simulink model "AcasXuClosedLoop_all_comp2017b"  (Simulink 9.0)
settings: Solver=VariableStepAuto  StartTime=0.0  StopTime=20  RelTol=1e-3
libraries: neural x14040, simulink x2
(part 38 of 534)

== system AcasXuClosedLoop_all_comp2017b/networks/Networks_m_9/AcasXu_3_9/Process Output 1 ==
  a : Inport  PortDimensions=5
      mask Display: e = 0.06;c1 = [120 230 180]/255;c2 = ([120 230 180]+0)/(256+128);c3 = ([120 230 180]+128)/(256+128);patch([0 1 1 0],[0 0 1 1],c1);patch([0 1 1 1-e 1-e 0+e],[0 0 1 1-e 0+e 0+e],c2);patch([0 0 1 1-e 0+e 0+e],[0 1 1 1-e 1-e 0+e],c3);plot([0 1 1 0 0],[0 0 1 1 0]);
  y : Outport  PortDimensions=5
      mask Display: e = 0.06;c1 = [120 230 180]/255;c2 = ([120 230 180]+0)/(256+128);c3 = ([120 230 180]+128)/(256+128);patch([0 1 1 0],[0 0 1 1],c1);patch([0 1 1 1-e 1-e 0+e],[0 0 1 1-e 0+e 0+e],c2);patch([0 0 1 1-e 0+e 0+e],[0 1 1 1-e 1-e 0+e],c3);plot([0 1 1 0 0],[0 0 1 1 0]);
  wires:
  a/1 -> y/1

== system AcasXuClosedLoop_all_comp2017b/networks/Networks_m_9/AcasXu_4_9/Layer 1 ==
  "p{1}" : Inport  PortDimensions=5
      mask Display: e = 0.06;c1 = [120 230 180]/255;c2 = ([120 230 180]+0)/(256+128);c3 = ([120 230 180]+128)/(256+128);patch([0 1 1 0],[0 0 1 1],c1);patch([0 1 1 1-e 1-e 0+e],[0 0 1 1-e 0+e 0+e],c2);patch([0 0 1 1-e 0+e 0+e],[0 1 1 1-e 1-e 0+e],c3);plot([0 1 1 0 0],[0 0 1 1 0]);
  Delays 1 : Subsystem
      mask Display: e = 0.06;c1 = [120 150 230]/255;c2 = ([120 150 230]+0)/383;c3 = ([120 150 230]+128)/383;patch([0 1 1 0],[0 0 1 1],c1);patch([0 1 1 1-e 1-e 0+e],[0 0 1 1-e 0+e 0+e],c2);patch([0 0 1 1-e 0+e 0+e],[0 1 1 1-e 1-e 0+e],c3);plot([0 1 1 0 0],[0 0 1 1 0]);disp('0');
  "IW{1,1}" : Subsystem
      mask Display: e = 0.06;c1 = [120 150 230]/255;c2 = ([120 150 230]+0)/383;c3 = ([120 150 230]+128)/383;patch([0 1 1 0],[0 0 1 1],c1);patch([0 1 1 1-e 1-e 0+e],[0 0 1 1-e 0+e 0+e],c2);patch([0 0 1 1-e 0+e 0+e],[0 1 1 1-e 1-e 0+e],c3);plot([0 1 1 0 0],[0 0 1 1 0]);disp('W');
  "b{1}" : Constant  Value=[-0.6222280000000000033111291486420668661594390869140625;-0.7286479999999999623838675688602961599826812744140625;-0.1096229999999999982218668037603492848575115203857421875;0.57803999999999999825917029738775454461574554443359375;0.1547759999999999969144681699617649428546428680419921875;-0.59774099999999996679633795793051831424236297607421875;-0.414026999999999978374631837141350843012332916259765625;-0.057743500000000003158362460453645326197147369384765625;-0.384350000000000024957813593573519028723239898681640625;-0.124423000000000005815792292196420021355152130126953125;-0.1039690000000000058566484995026257820427417755126953125;-0.40556999999999998607336237910203635692596435546875;0.13983000000000000984101689027738757431507110595703125;-0.321711000000000024723334490772685967385768890380859375;-0.27227699999999999125321892279316671192646026611328125;-0.0582171999999999967290165159283787943422794342041015625;0.0781797999999999937426053975286777131259441375732421875;0.1547659999999999869135791641383548267185688018798828125;-0.259782000000000012907008795082219876348972320556640625;-0.219667000000000001147526518252561800181865692138671875;-0.0356213999999999975099029825287288986146450042724609375;-0.287955000000000016502355038028326816856861114501953125;-0.041210900000000001697220142204969306476414203643798828125;-0.32555800000000001404032445861957967281341552734375;-0.509692999999999951654672258882783353328704833984375;-0.481935999999999975518250039385748095810413360595703125;0.05832880000000000009219291996487299911677837371826171875;-0.0853672000000000041897152414094307459890842437744140625;-0.1696100000000000107558406625685165636241436004638671875;0.2366870000000000084927620491725974716246128082275390625;-0.0724772999999999945064388384707854129374027252197265625;0.04211489999999999678248485679432633332908153533935546875;0.12322800000000000419930756834219209849834442138671875;0.1397259999999999890984980766006628982722759246826171875;-0.338374000000000008103739901343942619860172271728515625;-0.049813900000000001233591007121503935195505619049072265625;-0.490978999999999998760102926098625175654888153076171875;-0.03533540000000000291802138008279143832623958587646484375;-0.31175900000000000833466629046597518026828765869140625;0.0022653399999999998017108371328731664107181131839752197265625;-0.5714989999999999792379412610898725688457489013671875;0.072080599999999994675903280949569307267665863037109375;-0.05229720000000000201811900524262455292046070098876953125;0.2396560000000000079101170058493153192102909088134765625;0.9467330000000000467252903035841882228851318359375;-0.06532010000000000593534110748805687762796878814697265625;-0.475136999999999976029840809133020229637622833251953125;-0.52941099999999996494892684495425783097743988037109375;-0.39539000000000001922018100231071002781391143798828125;-0.1320530000000000037108094375071232207119464874267578125]
      mask Display: e = 0.06;c1 = [120 150 230]/255;c2 = ([120 150 230]+0)/383;c3 = ([120 150 230]+128)/383;patch([0 1 1 0],[0 0 1 1],c1);patch([0 1 1 1-e 1-e 0+e],[0 0 1 1-e 0+e 0+e],c2);patch([0 0 1 1-e 0+e 0+e],[0 1 1 1-e 1-e 0+e],c3);plot([0 1 1 0 0],[0 0 1 1 0]);disp('b');
  netsum : Sum  Inputs=2  SaturateOnIntegerOverflow=on  InputSameDT=on  OutDataTypeStr=Inherit: Same as first input
      mask Display: e = 0.06;c1 = [120 150 230]/255;c2 = ([120 150 230]+0)/383;c3 = ([120 150 230]+128)/383;patch([0 1 1 0],[0 0 1 1],c1);patch([0 1 1 1-e 1-e 0+e],[0 0 1 1-e 0+e 0+e],c2);patch([0 0 1 1-e 0+e 0+e],[0 1 1 1-e 1-e 0+e],c3);plot([0 1 1 0 0],[0 0 1 1 0]);disp('+');
  poslin : neural/Transfer Functions/poslin
      settings: SourceProductName=Deep Learning Toolbox  SourceProductBaseCode=NN
  "a{1}" : Outport  InitialOutput=[0;0;0;0;0;0;0;0;0;0;0;0;0;0;0;0;0;0;0;0;0;0;0;0;0;0;0;0;0;0;0;0;0;0;0;0;0;0;0;0;0;0;0;0;0;0;0;0;0;0]
      mask Display: e = 0.06;c1 = [120 230 180]/255;c2 = ([120 230 180]+0)/(256+128);c3 = ([120 230 180]+128)/(256+128);patch([0 1 1 0],[0 0 1 1],c1);patch([0 1 1 1-e 1-e 0+e],[0 0 1 1-e 0+e 0+e],c2);patch([0 0 1 1-e 0+e 0+e],[0 1 1 1-e 1-e 0+e],c3);plot([0 1 1 0 0],[0 0 1 1 0]);
  wires:
  poslin/1 -> "a{1}"/1
  netsum/1 -> poslin/1
  "b{1}"/1 -> netsum/2
  "IW{1,1}"/1("iz{1,1}") -> netsum/1
  Delays 1/1("pd{1,1}") -> "IW{1,1}"/1("pd{1,1}")
  "p{1}"/1 -> Delays 1/1("p{1}")

== system AcasXuClosedLoop_all_comp2017b/networks/Networks_m_9/AcasXu_4_9/Layer 2 ==
  "a{1} " : Inport  PortDimensions=50
      mask Display: e = 0.06;c1 = [120 230 180]/255;c2 = ([120 230 180]+0)/(256+128);c3 = ([120 230 180]+128)/(256+128);patch([0 1 1 0],[0 0 1 1],c1);patch([0 1 1 1-e 1-e 0+e],[0 0 1 1-e 0+e 0+e],c2);patch([0 0 1 1-e 0+e 0+e],[0 1 1 1-e 1-e 0+e],c3);plot([0 1 1 0 0],[0 0 1 1 0]);
  Delays 1 : Subsystem
      mask Display: e = 0.06;c1 = [120 150 230]/255;c2 = ([120 150 230]+0)/383;c3 = ([120 150 230]+128)/383;patch([0 1 1 0],[0 0 1 1],c1);patch([0 1 1 1-e 1-e 0+e],[0 0 1 1-e 0+e 0+e],c2);patch([0 0 1 1-e 0+e 0+e],[0 1 1 1-e 1-e 0+e],c3);plot([0 1 1 0 0],[0 0 1 1 0]);disp('0');
  "LW{2,1}" : Subsystem
      mask Display: e = 0.06;c1 = [120 150 230]/255;c2 = ([120 150 230]+0)/383;c3 = ([120 150 230]+128)/383;patch([0 1 1 0],[0 0 1 1],c1);patch([0 1 1 1-e 1-e 0+e],[0 0 1 1-e 0+e 0+e],c2);patch([0 0 1 1-e 0+e 0+e],[0 1 1 1-e 1-e 0+e],c3);plot([0 1 1 0 0],[0 0 1 1 0]);disp('W');
  "b{2}" : Constant  Value=[1.02522000000000002017941369558684527873992919921875;-0.562000999999999972800424075103364884853363037109375;-0.00946180999999999931826533128287337603978812694549560546875;0.17765500000000000735411731511703692376613616943359375;0.79179600000000005532996283363900147378444671630859375;-0.5709340000000000525659515915322117507457733154296875;-0.0059997399999999999342659151579937315545976161956787109375;-0.32771099999999997454125377771561034023761749267578125;-0.7948239999999999749746848465292714536190032958984375;0.76970400000000005480416120917652733623981475830078125;-0.0251702000000000002122302333873449242673814296722412109375;1.61854999999999993320898283855058252811431884765625;-0.3540360000000000173514536072616465389728546142578125;-0.00907804000000000076153749972718287608586251735687255859375;-0.0709912000000000042998493654522462747991085052490234375;0.368391999999999997239541471572010777890682220458984375;0.272660999999999986709298127607326023280620574951171875;-0.284345000000000014406253967536031268537044525146484375;-0.352752000000000009993783578465809114277362823486328125;0.96926900000000004720135393654345534741878509521484375;2.320460000000000189146476259338669478893280029296875;0.4902889999999999748325762993772514164447784423828125;-0.342196000000000000174082970261224545538425445556640625;0.398270999999999986140863938999245874583721160888671875;-0.016967400000000000537259126076605753041803836822509765625;0.331542999999999976612485852456302382051944732666015625;-0.06554050000000000153477230924181640148162841796875;0.4595569999999999932782657197094522416591644287109375;-1.162080000000000001847411112976260483264923095703125;-0.400434000000000012153833495176513679325580596923828125;0.55777299999999996327204598856042139232158660888671875;-0.291293000000000024130031306413002312183380126953125;-0.0113866000000000001934896687316722818650305271148681640625;-0.53071699999999999430855268656159751117229461669921875;-0.2219279999999999863913302533546811901032924652099609375;-0.015296099999999999974331643670666380785405635833740234375;-0.0083213399999999999867572597622711327858269214630126953125;-0.2919359999999999732978039901354350149631500244140625;-0.50425200000000003353051170051912777125835418701171875;-0.31965399999999999369748593380791135132312774658203125;1.0295199999999999906918901615426875650882720947265625;-0.75094000000000005190514684727531857788562774658203125;-0.45472400000000001707434194031520746648311614990234375;-0.01299699999999999987243537447056951350532472133636474609375;0.1546980000000000021742607714259065687656402587890625;-0.67860200000000003850431085083982907235622406005859375;-0.57151099999999999123900806807796470820903778076171875;-0.019285099999999999464961319972644560039043426513671875;-0.4202799999999999869260136620141565799713134765625;-0.52828500000000000458300064565264619886875152587890625]
      mask Display: e = 0.06;c1 = [120 150 230]/255;c2 = ([120 150 230]+0)/383;c3 = ([120 150 230]+128)/383;patch([0 1 1 0],[0 0 1 1],c1);patch([0 1 1 1-e 1-e 0+e],[0 0 1 1-e 0+e 0+e],c2);patch([0 0 1 1-e 0+e 0+e],[0 1 1 1-e 1-e 0+e],c3);plot([0 1 1 0 0],[0 0 1 1 0]);disp('b');
  netsum : Sum  Inputs=2  SaturateOnIntegerOverflow=on  InputSameDT=on  OutDataTypeStr=Inherit: Same as first input
      mask Display: e = 0.06;c1 = [120 150 230]/255;c2 = ([120 150 230]+0)/383;c3 = ([120 150 230]+128)/383;patch([0 1 1 0],[0 0 1 1],c1);patch([0 1 1 1-e 1-e 0+e],[0 0 1 1-e 0+e 0+e],c2);patch([0 0 1 1-e 0+e 0+e],[0 1 1 1-e 1-e 0+e],c3);plot([0 1 1 0 0],[0 0 1 1 0]);disp('+');
  poslin : neural/Transfer Functions/poslin
      settings: SourceProductName=Deep Learning Toolbox  SourceProductBaseCode=NN
  "a{2}" : Outport  InitialOutput=[0;0;0;0;0;0;0;0;0;0;0;0;0;0;0;0;0;0;0;0;0;0;0;0;0;0;0;0;0;0;0;0;0;0;0;0;0;0;0;0;0;0;0;0;0;0;0;0;0;0]
      mask Display: e = 0.06;c1 = [120 230 180]/255;c2 = ([120 230 180]+0)/(256+128);c3 = ([120 230 180]+128)/(256+128);patch([0 1 1 0],[0 0 1 1],c1);patch([0 1 1 1-e 1-e 0+e],[0 0 1 1-e 0+e 0+e],c2);patch([0 0 1 1-e 0+e 0+e],[0 1 1 1-e 1-e 0+e],c3);plot([0 1 1 0 0],[0 0 1 1 0]);
  wires:
  poslin/1 -> "a{2}"/1
  netsum/1 -> poslin/1
  "b{2}"/1 -> netsum/2
  "LW{2,1}"/1("lz{2,1}") -> netsum/1
  Delays 1/1("pd{2,1}") -> "LW{2,1}"/1("ad{2,1}")
  "a{1} "/1 -> Delays 1/1("p{2}")

== system AcasXuClosedLoop_all_comp2017b/networks/Networks_m_9/AcasXu_4_9/Layer 3 ==
  "a{2} " : Inport  PortDimensions=50
      mask Display: e = 0.06;c1 = [120 230 180]/255;c2 = ([120 230 180]+0)/(256+128);c3 = ([120 230 180]+128)/(256+128);patch([0 1 1 0],[0 0 1 1],c1);patch([0 1 1 1-e 1-e 0+e],[0 0 1 1-e 0+e 0+e],c2);patch([0 0 1 1-e 0+e 0+e],[0 1 1 1-e 1-e 0+e],c3);plot([0 1 1 0 0],[0 0 1 1 0]);
  Delays 1 : Subsystem
      mask Display: e = 0.06;c1 = [120 150 230]/255;c2 = ([120 150 230]+0)/383;c3 = ([120 150 230]+128)/383;patch([0 1 1 0],[0 0 1 1],c1);patch([0 1 1 1-e 1-e 0+e],[0 0 1 1-e 0+e 0+e],c2);patch([0 0 1 1-e 0+e 0+e],[0 1 1 1-e 1-e 0+e],c3);plot([0 1 1 0 0],[0 0 1 1 0]);disp('0');
  "LW{3,2}" : Subsystem
      mask Display: e = 0.06;c1 = [120 150 230]/255;c2 = ([120 150 230]+0)/383;c3 = ([120 150 230]+128)/383;patch([0 1 1 0],[0 0 1 1],c1);patch([0 1 1 1-e 1-e 0+e],[0 0 1 1-e 0+e 0+e],c2);patch([0 0 1 1-e 0+e 0+e],[0 1 1 1-e 1-e 0+e],c3);plot([0 1 1 0 0],[0 0 1 1 0]);disp('W');
  "b{3}" : Constant  Value=[-0.1822640000000000093383079047271166928112506866455078125;0.62188699999999996759214582198183052241802215576171875;1.226879999999999970583530739531852304935455322265625;0.387351999999999974111375422580749727785587310791015625;-2.192489999999999827906549398903734982013702392578125;-0.341052999999999995051069845430902205407619476318359375;-2.135260000000000157882595885894261300563812255859375;-0.65622400000000002950883981611696071922779083251953125;-1.2105399999999999494093572138808667659759521484375;0.293040000000000022684076839141198433935642242431640625;-1.134489999999999998436805981327779591083526611328125;-2.153630000000000155324642037157900631427764892578125;-0.365439999999999987068122209166176617145538330078125;-0.97034399999999998431832182177458889782428741455078125;0.280422000000000004593658786689047701656818389892578125;-0.5748100000000000431299440606380812823772430419921875;-0.632265999999999994685140336514450609683990478515625;0.8990700000000000358113538823090493679046630859375;-2.217560000000000197672989088459871709346771240234375;1.2218299999999999716493448431720025837421417236328125;-1.4797000000000000152766688188421539962291717529296875;-0.47529399999999999426592012241599150002002716064453125;-0.89124099999999994992094798362813889980316162109375;-3.7400000000000002131628207280300557613372802734375;-1.6563499999999999889865875957184471189975738525390625;0.34078900000000000858335624798201024532318115234375;2.00767000000000006565414878423325717449188232421875;-0.073932100000000000594724269831203855574131011962890625;-2.95357000000000002870592652470804750919342041015625;-0.484140999999999988023802188763511367142200469970703125;0.21756100000000000438404867963981814682483673095703125;-0.276013999999999981582732289098203182220458984375;-2.63429000000000002046363078989088535308837890625;-0.783528000000000002245315045001916587352752685546875;-3.292660000000000142250655699172057211399078369140625;1.0612299999999998956212721168412826955318450927734375;0.51146100000000005447731155072688125073909759521484375;0.79009099999999998775734866285347379744052886962890625;0.90034000000000002916777930295211263000965118408203125;-0.99270000000000002682298827494378201663494110107421875;-1.1930099999999999038635678516584448516368865966796875;0.65531600000000000960653778747655451297760009765625;0.27336199999999999388222704510553739964962005615234375;-1.218409999999999993036681189551018178462982177734375;1.033630000000000048743231673142872750759124755859375;0.165285999999999988485654967007576487958431243896484375;0.333407999999999982154719191385083831846714019775390625;0.07858579999999999732285260733988252468407154083251953125;-0.11880100000000000382005538313023862428963184356689453125;0.458838000000000023614887822986929677426815032958984375]
      mask Display: e = 0.06;c1 = [120 150 230]/255;c2 = ([120 150 230]+0)/383;c3 = ([120 150 230]+128)/383;patch([0 1 1 0],[0 0 1 1],c1);patch([0 1 1 1-e 1-e 0+e],[0 0 1 1-e 0+e 0+e],c2);patch([0 0 1 1-e 0+e 0+e],[0 1 1 1-e 1-e 0+e],c3);plot([0 1 1 0 0],[0 0 1 1 0]);disp('b');
  netsum : Sum  Inputs=2  SaturateOnIntegerOverflow=on  InputSameDT=on  OutDataTypeStr=Inherit: Same as first input
      mask Display: e = 0.06;c1 = [120 150 230]/255;c2 = ([120 150 230]+0)/383;c3 = ([120 150 230]+128)/383;patch([0 1 1 0],[0 0 1 1],c1);patch([0 1 1 1-e 1-e 0+e],[0 0 1 1-e 0+e 0+e],c2);patch([0 0 1 1-e 0+e 0+e],[0 1 1 1-e 1-e 0+e],c3);plot([0 1 1 0 0],[0 0 1 1 0]);disp('+');
  poslin : neural/Transfer Functions/poslin
      settings: SourceProductName=Deep Learning Toolbox  SourceProductBaseCode=NN
  "a{3}" : Outport  InitialOutput=[0;0;0;0;0;0;0;0;0;0;0;0;0;0;0;0;0;0;0;0;0;0;0;0;0;0;0;0;0;0;0;0;0;0;0;0;0;0;0;0;0;0;0;0;0;0;0;0;0;0]
      mask Display: e = 0.06;c1 = [120 230 180]/255;c2 = ([120 230 180]+0)/(256+128);c3 = ([120 230 180]+128)/(256+128);patch([0 1 1 0],[0 0 1 1],c1);patch([0 1 1 1-e 1-e 0+e],[0 0 1 1-e 0+e 0+e],c2);patch([0 0 1 1-e 0+e 0+e],[0 1 1 1-e 1-e 0+e],c3);plot([0 1 1 0 0],[0 0 1 1 0]);
  wires:
  poslin/1 -> "a{3}"/1
  netsum/1 -> poslin/1
  "b{3}"/1 -> netsum/2
  "LW{3,2}"/1("lz{3,2}") -> netsum/1
  Delays 1/1("pd{3,2}") -> "LW{3,2}"/1("ad{3,2}")
  "a{2} "/1 -> Delays 1/1("p{3}")

== system AcasXuClosedLoop_all_comp2017b/networks/Networks_m_9/AcasXu_4_9/Layer 4 ==
  "a{3} " : Inport  PortDimensions=50
      mask Display: e = 0.06;c1 = [120 230 180]/255;c2 = ([120 230 180]+0)/(256+128);c3 = ([120 230 180]+128)/(256+128);patch([0 1 1 0],[0 0 1 1],c1);patch([0 1 1 1-e 1-e 0+e],[0 0 1 1-e 0+e 0+e],c2);patch([0 0 1 1-e 0+e 0+e],[0 1 1 1-e 1-e 0+e],c3);plot([0 1 1 0 0],[0 0 1 1 0]);
  Delays 1 : Subsystem
      mask Display: e = 0.06;c1 = [120 150 230]/255;c2 = ([120 150 230]+0)/383;c3 = ([120 150 230]+128)/383;patch([0 1 1 0],[0 0 1 1],c1);patch([0 1 1 1-e 1-e 0+e],[0 0 1 1-e 0+e 0+e],c2);patch([0 0 1 1-e 0+e 0+e],[0 1 1 1-e 1-e 0+e],c3);plot([0 1 1 0 0],[0 0 1 1 0]);disp('0');
  "LW{4,3}" : Subsystem
      mask Display: e = 0.06;c1 = [120 150 230]/255;c2 = ([120 150 230]+0)/383;c3 = ([120 150 230]+128)/383;patch([0 1 1 0],[0 0 1 1],c1);patch([0 1 1 1-e 1-e 0+e],[0 0 1 1-e 0+e 0+e],c2);patch([0 0 1 1-e 0+e 0+e],[0 1 1 1-e 1-e 0+e],c3);plot([0 1 1 0 0],[0 0 1 1 0]);disp('W');
  "b{4}" : Constant  Value=[-0.89365000000000005542233338928781449794769287109375;0.1854739999999999999769073610877967439591884613037109375;-1.8181799999999999073452272568829357624053955078125;2.8344100000000000960653778747655451297760009765625;-1.0596099999999999408117901111836545169353485107421875;-5.882780000000000342197381542064249515533447265625;-2.30323000000000011056044968427158892154693603515625;-0.78521300000000004981615120414062403142452239990234375;-2.105259999999999909192638369859196245670318603515625;-2.711160000000000014352963262354023754596710205078125;1.9786099999999999798916405779891647398471832275390625;-1.0117499999999999271693695845897309482097625732421875;2.79769999999999985362819643341936171054840087890625;0.2040669999999999983941734171821735799312591552734375;-0.380328999999999972647657386914943344891071319580078125;-0.006045140000000000062019278601610494661144912242889404296875;0.06022650000000000225508500761861796490848064422607421875;-0.05921819999999999861728383621084503829479217529296875;5.17250999999999994116706147906370460987091064453125;1.5604800000000000892441676114685833454132080078125;1.4627499999999999946709294817992486059665679931640625;-2.630640000000000089386276158620603382587432861328125;1.65083000000000001961097950697876513004302978515625;-2.36413999999999990819787853979505598545074462890625;0.146766000000000007563727422166266478598117828369140625;-1.598829999999999973425701682572253048419952392578125;-0.065557699999999996531840906754950992763042449951171875;-2.61073999999999983856469043530523777008056640625;2.0779399999999998982502802391536533832550048828125;-1.2690300000000001023892082230304367840290069580078125;-0.1732309999999999960973440238376497291028499603271484375;3.5928100000000000591171556152403354644775390625;-2.93836999999999992638777257525362074375152587890625;-1.4845600000000001017497197608463466167449951171875;1.0714900000000000535038680027355439960956573486328125;-5.20971999999999990649257597397081553936004638671875;1.764180000000000081428197518107481300830841064453125;-2.023140000000000160440549734630621969699859619140625;-0.54592300000000004711608880825224332511425018310546875;-1.1615599999999999258903926602215506136417388916015625;-0.343324000000000018051338201985345222055912017822265625;-0.0765071999999999974306774674914777278900146484375;-2.868729999999999780158077555825002491474151611328125;-2.16922000000000014807710613240487873554229736328125;0.034253999999999999725996957522511365823447704315185546875;-5.417989999999999639612724422477185726165771484375;-2.7816700000000000869704308570362627506256103515625;-2.69573000000000018161472326028160750865936279296875;-1.645869999999999944151340969256125390529632568359375;-0.041442600000000003046540797413399559445679187774658203125]
      mask Display: e = 0.06;c1 = [120 150 230]/255;c2 = ([120 150 230]+0)/383;c3 = ([120 150 230]+128)/383;patch([0 1 1 0],[0 0 1 1],c1);patch([0 1 1 1-e 1-e 0+e],[0 0 1 1-e 0+e 0+e],c2);patch([0 0 1 1-e 0+e 0+e],[0 1 1 1-e 1-e 0+e],c3);plot([0 1 1 0 0],[0 0 1 1 0]);disp('b');
  netsum : Sum  Inputs=2  SaturateOnIntegerOverflow=on  InputSameDT=on  OutDataTypeStr=Inherit: Same as first input
      mask Display: e = 0.06;c1 = [120 150 230]/255;c2 = ([120 150 230]+0)/383;c3 = ([120 150 230]+128)/383;patch([0 1 1 0],[0 0 1 1],c1);patch([0 1 1 1-e 1-e 0+e],[0 0 1 1-e 0+e 0+e],c2);patch([0 0 1 1-e 0+e 0+e],[0 1 1 1-e 1-e 0+e],c3);plot([0 1 1 0 0],[0 0 1 1 0]);disp('+');
  poslin : neural/Transfer Functions/poslin
      settings: SourceProductName=Deep Learning Toolbox  SourceProductBaseCode=NN
  "a{4}" : Outport  InitialOutput=[0;0;0;0;0;0;0;0;0;0;0;0;0;0;0;0;0;0;0;0;0;0;0;0;0;0;0;0;0;0;0;0;0;0;0;0;0;0;0;0;0;0;0;0;0;0;0;0;0;0]
      mask Display: e = 0.06;c1 = [120 230 180]/255;c2 = ([120 230 180]+0)/(256+128);c3 = ([120 230 180]+128)/(256+128);patch([0 1 1 0],[0 0 1 1],c1);patch([0 1 1 1-e 1-e 0+e],[0 0 1 1-e 0+e 0+e],c2);patch([0 0 1 1-e 0+e 0+e],[0 1 1 1-e 1-e 0+e],c3);plot([0 1 1 0 0],[0 0 1 1 0]);
  wires:
  poslin/1 -> "a{4}"/1
  netsum/1 -> poslin/1
  "b{4}"/1 -> netsum/2
  "LW{4,3}"/1("lz{4,3}") -> netsum/1
  Delays 1/1("pd{4,3}") -> "LW{4,3}"/1("ad{4,3}")
  "a{3} "/1 -> Delays 1/1("p{4}")

== system AcasXuClosedLoop_all_comp2017b/networks/Networks_m_9/AcasXu_4_9/Layer 5 ==
  "a{4} " : Inport  PortDimensions=50
      mask Display: e = 0.06;c1 = [120 230 180]/255;c2 = ([120 230 180]+0)/(256+128);c3 = ([120 230 180]+128)/(256+128);patch([0 1 1 0],[0 0 1 1],c1);patch([0 1 1 1-e 1-e 0+e],[0 0 1 1-e 0+e 0+e],c2);patch([0 0 1 1-e 0+e 0+e],[0 1 1 1-e 1-e 0+e],c3);plot([0 1 1 0 0],[0 0 1 1 0]);
  Delays 1 : Subsystem
      mask Display: e = 0.06;c1 = [120 150 230]/255;c2 = ([120 150 230]+0)/383;c3 = ([120 150 230]+128)/383;patch([0 1 1 0],[0 0 1 1],c1);patch([0 1 1 1-e 1-e 0+e],[0 0 1 1-e 0+e 0+e],c2);patch([0 0 1 1-e 0+e 0+e],[0 1 1 1-e 1-e 0+e],c3);plot([0 1 1 0 0],[0 0 1 1 0]);disp('0');
  "LW{5,4}" : Subsystem
      mask Display: e = 0.06;c1 = [120 150 230]/255;c2 = ([120 150 230]+0)/383;c3 = ([120 150 230]+128)/383;patch([0 1 1 0],[0 0 1 1],c1);patch([0 1 1 1-e 1-e 0+e],[0 0 1 1-e 0+e 0+e],c2);patch([0 0 1 1-e 0+e 0+e],[0 1 1 1-e 1-e 0+e],c3);plot([0 1 1 0 0],[0 0 1 1 0]);disp('W');
  "b{5}" : Constant  Value=[-0.0191056999999999997663646666978820576332509517669677734375;1.3559200000000000141398004416259936988353729248046875;-0.71357999999999999207744849627488292753696441650390625;-8.3485700000000004905587047687731683254241943359375;-1.559309999999999973852027324028313159942626953125;2.3629400000000000403588273911736905574798583984375;0.51666100000000003689137884066440165042877197265625;0.468301000000000022804869104220415465533733367919921875;-0.261516000000000026215474235868896357715129852294921875;3.70849999999999990762944435118697583675384521484375;-0.89031599999999999628386149197467602789402008056640625;-0.026237400000000000999111904320670873858034610748291015625;0.19159599999999998853894567218958400189876556396484375;1.3666899999999999604227696181624196469783782958984375;2.403910000000000213304929275182075798511505126953125;2.186739999999999906066250332514755427837371826171875;-0.76852200000000003843325657726381905376911163330078125;0.315346999999999988428811548146768473088741302490234375;-0.87672000000000005481837206389172933995723724365234375;-1.57667000000000001591615728102624416351318359375;0.6877590000000000092228447101661004126071929931640625;-5.07626000000000043854697651113383471965789794921875;0.9768879999999999785842419441905803978443145751953125;-0.9757780000000000342197381542064249515533447265625;1.7846100000000000296296320811961777508258819580078125;4.19756999999999980133225108147598803043365478515625;-1.7266799999999999926103555480949580669403076171875;-1.93334999999999990194510246510617434978485107421875;-1.4522500000000000408562073062057606875896453857421875;-0.29601800000000000334665628543007187545299530029296875;-3.774789999999999867696942601469345390796661376953125;-0.189578999999999997516653138518449850380420684814453125;1.0545700000000000073896444519050419330596923828125;-0.11671499999999999930277994053540169261395931243896484375;2.40486999999999984112264428404159843921661376953125;4.52629000000000036862957131233997642993927001953125;2.59363000000000010203393685515038669109344482421875;3.37394000000000016115109247039072215557098388671875;1.9498800000000000576250158701441250741481781005859375;-2.4771499999999999630517777404747903347015380859375;-5.73190000000000043911541069974191486835479736328125;1.1379099999999999770494696349487639963626861572265625;-2.4970400000000001483613232267089188098907470703125;2.980239999999999778168557895696721971035003662109375;-0.66089600000000003898747991115669719874858856201171875;3.55039000000000015688783605583012104034423828125;-3.1092800000000000437694325228221714496612548828125;-0.27077600000000001667643800828955136239528656005859375;2.273099999999999898392388786305673420429229736328125;-1.494310000000000027142732506035827100276947021484375]
      mask Display: e = 0.06;c1 = [120 150 230]/255;c2 = ([120 150 230]+0)/383;c3 = ([120 150 230]+128)/383;patch([0 1 1 0],[0 0 1 1],c1);patch([0 1 1 1-e 1-e 0+e],[0 0 1 1-e 0+e 0+e],c2);patch([0 0 1 1-e 0+e 0+e],[0 1 1 1-e 1-e 0+e],c3);plot([0 1 1 0 0],[0 0 1 1 0]);disp('b');
  netsum : Sum  Inputs=2  SaturateOnIntegerOverflow=on  InputSameDT=on  OutDataTypeStr=Inherit: Same as first input
      mask Display: e = 0.06;c1 = [120 150 230]/255;c2 = ([120 150 230]+0)/383;c3 = ([120 150 230]+128)/383;patch([0 1 1 0],[0 0 1 1],c1);patch([0 1 1 1-e 1-e 0+e],[0 0 1 1-e 0+e 0+e],c2);patch([0 0 1 1-e 0+e 0+e],[0 1 1 1-e 1-e 0+e],c3);plot([0 1 1 0 0],[0 0 1 1 0]);disp('+');
  poslin : neural/Transfer Functions/poslin
      settings: SourceProductName=Deep Learning Toolbox  SourceProductBaseCode=NN
  "a{5}" : Outport  InitialOutput=[0;0;0;0;0;0;0;0;0;0;0;0;0;0;0;0;0;0;0;0;0;0;0;0;0;0;0;0;0;0;0;0;0;0;0;0;0;0;0;0;0;0;0;0;0;0;0;0;0;0]
      mask Display: e = 0.06;c1 = [120 230 180]/255;c2 = ([120 230 180]+0)/(256+128);c3 = ([120 230 180]+128)/(256+128);patch([0 1 1 0],[0 0 1 1],c1);patch([0 1 1 1-e 1-e 0+e],[0 0 1 1-e 0+e 0+e],c2);patch([0 0 1 1-e 0+e 0+e],[0 1 1 1-e 1-e 0+e],c3);plot([0 1 1 0 0],[0 0 1 1 0]);
  wires:
  poslin/1 -> "a{5}"/1
  netsum/1 -> poslin/1
  "b{5}"/1 -> netsum/2
  "LW{5,4}"/1("lz{5,4}") -> netsum/1
  Delays 1/1("pd{5,4}") -> "LW{5,4}"/1("ad{5,4}")
  "a{4} "/1 -> Delays 1/1("p{5}")

== system AcasXuClosedLoop_all_comp2017b/networks/Networks_m_9/AcasXu_4_9/Layer 6 ==
  "a{5} " : Inport  PortDimensions=50
      mask Display: e = 0.06;c1 = [120 230 180]/255;c2 = ([120 230 180]+0)/(256+128);c3 = ([120 230 180]+128)/(256+128);patch([0 1 1 0],[0 0 1 1],c1);patch([0 1 1 1-e 1-e 0+e],[0 0 1 1-e 0+e 0+e],c2);patch([0 0 1 1-e 0+e 0+e],[0 1 1 1-e 1-e 0+e],c3);plot([0 1 1 0 0],[0 0 1 1 0]);
  Delays 1 : Subsystem
      mask Display: e = 0.06;c1 = [120 150 230]/255;c2 = ([120 150 230]+0)/383;c3 = ([120 150 230]+128)/383;patch([0 1 1 0],[0 0 1 1],c1);patch([0 1 1 1-e 1-e 0+e],[0 0 1 1-e 0+e 0+e],c2);patch([0 0 1 1-e 0+e 0+e],[0 1 1 1-e 1-e 0+e],c3);plot([0 1 1 0 0],[0 0 1 1 0]);disp('0');
  "LW{6,5}" : Subsystem
      mask Display: e = 0.06;c1 = [120 150 230]/255;c2 = ([120 150 230]+0)/383;c3 = ([120 150 230]+128)/383;patch([0 1 1 0],[0 0 1 1],c1);patch([0 1 1 1-e 1-e 0+e],[0 0 1 1-e 0+e 0+e],c2);patch([0 0 1 1-e 0+e 0+e],[0 1 1 1-e 1-e 0+e],c3);plot([0 1 1 0 0],[0 0 1 1 0]);disp('W');
  "b{6}" : Constant  Value=[3.47473000000000009634959496906958520412445068359375;-0.76460099999999997510968796632369048893451690673828125;-2.842560000000000197672989088459871709346771240234375;-5.66467000000000009407585821463726460933685302734375;0.121755000000000002113864638886298052966594696044921875;-0.2228239999999999942925654750069952569901943206787109375;1.6902399999999999646860260327230207622051239013671875;-2.8998200000000000642330633127130568027496337890625;2.301550000000000206767936106189154088497161865234375;0.8894349999999999756283841634285636246204376220703125;2.950919999999999987494447850622236728668212890625;3.1411899999999999266719896695576608180999755859375;3.9182100000000001926991899381391704082489013671875;5.5350500000000000255795384873636066913604736328125;-4.911590000000000344471118296496570110321044921875;3.93102999999999980218490236438810825347900390625;-2.903490000000000126334498418145813047885894775390625;-1.28774999999999995026200849679298698902130126953125;-2.450200000000000155608859131461940705776214599609375;-2.1671800000000001062971932697109878063201904296875;-6.09743000000000012761347534251399338245391845703125;-7.596199999999999619149093632586300373077392578125;-2.58457999999999987750243235495872795581817626953125;-0.2267530000000000101056940593480248935520648956298828125;-1.70751000000000008327560863108374178409576416015625;0.662668000000000034788172342814505100250244140625;3.66957000000000022055246517993509769439697265625;-2.662520000000000219841922444174997508525848388671875;1.6607600000000000139976918944739736616611480712890625;-2.701020000000000198525640371371991932392120361328125;4.69108000000000036067149267182685434818267822265625;-2.577240000000000197388771994155831634998321533203125;2.096670000000000033679725675028748810291290283203125;6.0103600000000003689137884066440165042877197265625;2.811970000000000080575546235195361077785491943359375;0.351059999999999983177900730879628099501132965087890625;1.62349999999999994315658113919198513031005859375;-2.54253000000000017877255231724120676517486572265625;0.0238302999999999987057464068129775114357471466064453125;0.12396400000000000474198458277896861545741558074951171875;2.445469999999999810569306646357290446758270263671875;-4.7998700000000003029754225281067192554473876953125;-2.496849999999999791810978422290645539760589599609375;1.4914099999999999024424823801382444798946380615234375;-4.76001000000000029643842935911379754543304443359375;0.66468899999999997429966924755717627704143524169921875;-3.36561999999999983401721692644059658050537109375;0.702080000000000037374547900981269776821136474609375;-2.528090000000000170388148035272024571895599365234375;-3.319780000000000175219838638440705835819244384765625]
      mask Display: e = 0.06;c1 = [120 150 230]/255;c2 = ([120 150 230]+0)/383;c3 = ([120 150 230]+128)/383;patch([0 1 1 0],[0 0 1 1],c1);patch([0 1 1 1-e 1-e 0+e],[0 0 1 1-e 0+e 0+e],c2);patch([0 0 1 1-e 0+e 0+e],[0 1 1 1-e 1-e 0+e],c3);plot([0 1 1 0 0],[0 0 1 1 0]);disp('b');
  netsum : Sum  Inputs=2  SaturateOnIntegerOverflow=on  InputSameDT=on  OutDataTypeStr=Inherit: Same as first input
      mask Display: e = 0.06;c1 = [120 150 230]/255;c2 = ([120 150 230]+0)/383;c3 = ([120 150 230]+128)/383;patch([0 1 1 0],[0 0 1 1],c1);patch([0 1 1 1-e 1-e 0+e],[0 0 1 1-e 0+e 0+e],c2);patch([0 0 1 1-e 0+e 0+e],[0 1 1 1-e 1-e 0+e],c3);plot([0 1 1 0 0],[0 0 1 1 0]);disp('+');
  poslin : neural/Transfer Functions/poslin
      settings: SourceProductName=Deep Learning Toolbox  SourceProductBaseCode=NN
  "a{6}" : Outport  InitialOutput=[0;0;0;0;0;0;0;0;0;0;0;0;0;0;0;0;0;0;0;0;0;0;0;0;0;0;0;0;0;0;0;0;0;0;0;0;0;0;0;0;0;0;0;0;0;0;0;0;0;0]
      mask Display: e = 0.06;c1 = [120 230 180]/255;c2 = ([120 230 180]+0)/(256+128);c3 = ([120 230 180]+128)/(256+128);patch([0 1 1 0],[0 0 1 1],c1);patch([0 1 1 1-e 1-e 0+e],[0 0 1 1-e 0+e 0+e],c2);patch([0 0 1 1-e 0+e 0+e],[0 1 1 1-e 1-e 0+e],c3);plot([0 1 1 0 0],[0 0 1 1 0]);
  wires:
  poslin/1 -> "a{6}"/1
  netsum/1 -> poslin/1
  "b{6}"/1 -> netsum/2
  "LW{6,5}"/1("lz{6,5}") -> netsum/1
  Delays 1/1("pd{6,5}") -> "LW{6,5}"/1("ad{6,5}")
  "a{5} "/1 -> Delays 1/1("p{6}")

== system AcasXuClosedLoop_all_comp2017b/networks/Networks_m_9/AcasXu_4_9/Layer 7 ==
  "a{6} " : Inport  PortDimensions=50
      mask Display: e = 0.06;c1 = [120 230 180]/255;c2 = ([120 230 180]+0)/(256+128);c3 = ([120 230 180]+128)/(256+128);patch([0 1 1 0],[0 0 1 1],c1);patch([0 1 1 1-e 1-e 0+e],[0 0 1 1-e 0+e 0+e],c2);patch([0 0 1 1-e 0+e 0+e],[0 1 1 1-e 1-e 0+e],c3);plot([0 1 1 0 0],[0 0 1 1 0]);
  Delays 1 : Subsystem
      mask Display: e = 0.06;c1 = [120 150 230]/255;c2 = ([120 150 230]+0)/383;c3 = ([120 150 230]+128)/383;patch([0 1 1 0],[0 0 1 1],c1);patch([0 1 1 1-e 1-e 0+e],[0 0 1 1-e 0+e 0+e],c2);patch([0 0 1 1-e 0+e 0+e],[0 1 1 1-e 1-e 0+e],c3);plot([0 1 1 0 0],[0 0 1 1 0]);disp('0');
  "LW{7,6}" : Subsystem
      mask Display: e = 0.06;c1 = [120 150 230]/255;c2 = ([120 150 230]+0)/383;c3 = ([120 150 230]+128)/383;patch([0 1 1 0],[0 0 1 1],c1);patch([0 1 1 1-e 1-e 0+e],[0 0 1 1-e 0+e 0+e],c2);patch([0 0 1 1-e 0+e 0+e],[0 1 1 1-e 1-e 0+e],c3);plot([0 1 1 0 0],[0 0 1 1 0]);disp('W');
  "b{7}" : Constant  Value=[-0.021089900000000001700772855883769807405769824981689453125;-0.018366500000000000880628903132674167864024639129638671875;0.018368400000000000005240252676230738870799541473388671875;-0.018527200000000000612576656067176372744143009185791015625;0.01852840000000000042490455598453991115093231201171875]
      mask Display: e = 0.06;c1 = [120 150 230]/255;c2 = ([120 150 230]+0)/383;c3 = ([120 150 230]+128)/383;patch([0 1 1 0],[0 0 1 1],c1);patch([0 1 1 1-e 1-e 0+e],[0 0 1 1-e 0+e 0+e],c2);patch([0 0 1 1-e 0+e 0+e],[0 1 1 1-e 1-e 0+e],c3);plot([0 1 1 0 0],[0 0 1 1 0]);disp('b');
  netsum : Sum  Inputs=2  SaturateOnIntegerOverflow=on  InputSameDT=on  OutDataTypeStr=Inherit: Same as first input
      mask Display: e = 0.06;c1 = [120 150 230]/255;c2 = ([120 150 230]+0)/383;c3 = ([120 150 230]+128)/383;patch([0 1 1 0],[0 0 1 1],c1);patch([0 1 1 1-e 1-e 0+e],[0 0 1 1-e 0+e 0+e],c2);patch([0 0 1 1-e 0+e 0+e],[0 1 1 1-e 1-e 0+e],c3);plot([0 1 1 0 0],[0 0 1 1 0]);disp('+');
  purelin : neural/Transfer Functions/purelin
      settings: SourceProductName=Deep Learning Toolbox
  "a{7}" : Outport  InitialOutput=[0;0;0;0;0]
      mask Display: e = 0.06;c1 = [120 230 180]/255;c2 = ([120 230 180]+0)/(256+128);c3 = ([120 230 180]+128)/(256+128);patch([0 1 1 0],[0 0 1 1],c1);patch([0 1 1 1-e 1-e 0+e],[0 0 1 1-e 0+e 0+e],c2);patch([0 0 1 1-e 0+e 0+e],[0 1 1 1-e 1-e 0+e],c3);plot([0 1 1 0 0],[0 0 1 1 0]);
  wires:
  purelin/1 -> "a{7}"/1
  netsum/1 -> purelin/1
  "b{7}"/1 -> netsum/2
  "LW{7,6}"/1("lz{7,6}") -> netsum/1
  Delays 1/1("pd{7,6}") -> "LW{7,6}"/1("ad{7,6}")
  "a{6} "/1 -> Delays 1/1("p{7}")

== system AcasXuClosedLoop_all_comp2017b/networks/Networks_m_9/AcasXu_4_9/Process Input 1 ==
  x : Inport  PortDimensions=5
      mask Display: e = 0.06;c1 = [120 230 180]/255;c2 = ([120 230 180]+0)/(256+128);c3 = ([120 230 180]+128)/(256+128);patch([0 1 1 0],[0 0 1 1],c1);patch([0 1 1 1-e 1-e 0+e],[0 0 1 1-e 0+e 0+e],c2);patch([0 0 1 1-e 0+e 0+e],[0 1 1 1-e 1-e 0+e],c3);plot([0 1 1 0 0],[0 0 1 1 0]);
  p : Outport  PortDimensions=5
      mask Display: e = 0.06;c1 = [120 230 180]/255;c2 = ([120 230 180]+0)/(256+128);c3 = ([120 230 180]+128)/(256+128);patch([0 1 1 0],[0 0 1 1],c1);patch([0 1 1 1-e 1-e 0+e],[0 0 1 1-e 0+e 0+e],c2);patch([0 0 1 1-e 0+e 0+e],[0 1 1 1-e 1-e 0+e],c3);plot([0 1 1 0 0],[0 0 1 1 0]);
  wires:
  x/1 -> p/1

== system AcasXuClosedLoop_all_comp2017b/networks/Networks_m_9/AcasXu_4_9/Process Output 1 ==
  a : Inport  PortDimensions=5
      mask Display: e = 0.06;c1 = [120 230 180]/255;c2 = ([120 230 180]+0)/(256+128);c3 = ([120 230 180]+128)/(256+128);patch([0 1 1 0],[0 0 1 1],c1);patch([0 1 1 1-e 1-e 0+e],[0 0 1 1-e 0+e 0+e],c2);patch([0 0 1 1-e 0+e 0+e],[0 1 1 1-e 1-e 0+e],c3);plot([0 1 1 0 0],[0 0 1 1 0]);
  y : Outport  PortDimensions=5
      mask Display: e = 0.06;c1 = [120 230 180]/255;c2 = ([120 230 180]+0)/(256+128);c3 = ([120 230 180]+128)/(256+128);patch([0 1 1 0],[0 0 1 1],c1);patch([0 1 1 1-e 1-e 0+e],[0 0 1 1-e 0+e 0+e],c2);patch([0 0 1 1-e 0+e 0+e],[0 1 1 1-e 1-e 0+e],c3);plot([0 1 1 0 0],[0 0 1 1 0]);
  wires:
  a/1 -> y/1

== system AcasXuClosedLoop_all_comp2017b/networks/Networks_m_9/AcasXu_5_9/Layer 1 ==
  "p{1}" : Inport  PortDimensions=5
      mask Display: e = 0.06;c1 = [120 230 180]/255;c2 = ([120 230 180]+0)/(256+128);c3 = ([120 230 180]+128)/(256+128);patch([0 1 1 0],[0 0 1 1],c1);patch([0 1 1 1-e 1-e 0+e],[0 0 1 1-e 0+e 0+e],c2);patch([0 0 1 1-e 0+e 0+e],[0 1 1 1-e 1-e 0+e],c3);plot([0 1 1 0 0],[0 0 1 1 0]);
  Delays 1 : Subsystem
      mask Display: e = 0.06;c1 = [120 150 230]/255;c2 = ([120 150 230]+0)/383;c3 = ([120 150 230]+128)/383;patch([0 1 1 0],[0 0 1 1],c1);patch([0 1 1 1-e 1-e 0+e],[0 0 1 1-e 0+e 0+e],c2);patch([0 0 1 1-e 0+e 0+e],[0 1 1 1-e 1-e 0+e],c3);plot([0 1 1 0 0],[0 0 1 1 0]);disp('0');
  "IW{1,1}" : Subsystem
      mask Display: e = 0.06;c1 = [120 150 230]/255;c2 = ([120 150 230]+0)/383;c3 = ([120 150 230]+128)/383;patch([0 1 1 0],[0 0 1 1],c1);patch([0 1 1 1-e 1-e 0+e],[0 0 1 1-e 0+e 0+e],c2);patch([0 0 1 1-e 0+e 0+e],[0 1 1 1-e 1-e 0+e],c3);plot([0 1 1 0 0],[0 0 1 1 0]);disp('W');
  "b{1}" : Constant  Value=[0.00080095100000000005006917103145269720698706805706024169921875;-0.38161699999999998400568301804014481604099273681640625;-0.538820999999999994400923242210410535335540771484375;0.033583200000000000551292345107867731712758541107177734375;0.030030199999999999949107376551182824186980724334716796875;-0.0093161200000000006504752292357807164080440998077392578125;-0.254778000000000004465761094252229668200016021728515625;-0.11311200000000000420730117411949322558939456939697265625;0.03660739999999999827462460189053672365844249725341796875;-0.0182462000000000006127987006721014040522277355194091796875;0.155247999999999997111643779135192744433879852294921875;0.0097140000000000004121147867408581078052520751953125;-0.40895599999999998619415464418125338852405548095703125;-0.0282319000000000007444267424716599634848535060882568359375;-0.1606740000000000112567732912793871946632862091064453125;-0.334274000000000015564438626824994571506977081298828125;-0.00852013000000000075839778901354293338954448699951171875;0.044436599999999999710542653019729186780750751495361328125;-0.376109000000000026631141736288554966449737548828125;0.53439000000000003165467887811246328055858612060546875;-0.52651499999999995527133478390169329941272735595703125;-0.1708280000000000076187944841876742430031299591064453125;-0.21827099999999999280220208675018511712551116943359375;-0.55273099999999997233857129685929976403713226318359375;-0.3421279999999999876791889619198627769947052001953125;-0.11433500000000000607069949865035596303641796112060546875;-0.49033300000000001883648792500025592744350433349609375;-0.1667889999999999928181892983047873713076114654541015625;0.1443319999999999880824219644637196324765682220458984375;-0.115534999999999998809840917601832188665866851806640625;-0.09580170000000000352091689137523644603788852691650390625;-0.07271270000000000510720354895966011099517345428466796875;-0.06675340000000000439950298414260032586753368377685546875;-0.0165737000000000002375433183487984933890402317047119140625;-0.030902800000000001101607693954065325669944286346435546875;-0.27864400000000000279243295153719373047351837158203125;-0.179914999999999991597832149636815302073955535888671875;-0.53926799999999996959587633682531304657459259033203125;-0.09856410000000000171116454339426127262413501739501953125;-0.1871460000000000067910121970271575264632701873779296875;-0.1604750000000000065281113847959204576909542083740234375;-0.1330240000000000033519853559482726268470287322998046875;-0.1398989999999999955804241835721768438816070556640625;-0.34521699999999999608490952596184797585010528564453125;0.0267532000000000012851497643850962049327790737152099609375;-0.45369599999999998818367430430953390896320343017578125;0.0662925000000000042010839251815923489630222320556640625;-0.0269639999999999983859577667999474215321242809295654296875;-0.444176999999999988499865821722778491675853729248046875;0.0302394999999999990192289800461367121897637844085693359375]
      mask Display: e = 0.06;c1 = [120 150 230]/255;c2 = ([120 150 230]+0)/383;c3 = ([120 150 230]+128)/383;patch([0 1 1 0],[0 0 1 1],c1);patch([0 1 1 1-e 1-e 0+e],[0 0 1 1-e 0+e 0+e],c2);patch([0 0 1 1-e 0+e 0+e],[0 1 1 1-e 1-e 0+e],c3);plot([0 1 1 0 0],[0 0 1 1 0]);disp('b');
  netsum : Sum  Inputs=2  SaturateOnIntegerOverflow=on  InputSameDT=on  OutDataTypeStr=Inherit: Same as first input
      mask Display: e = 0.06;c1 = [120 150 230]/255;c2 = ([120 150 230]+0)/383;c3 = ([120 150 230]+128)/383;patch([0 1 1 0],[0 0 1 1],c1);patch([0 1 1 1-e 1-e 0+e],[0 0 1 1-e 0+e 0+e],c2);patch([0 0 1 1-e 0+e 0+e],[0 1 1 1-e 1-e 0+e],c3);plot([0 1 1 0 0],[0 0 1 1 0]);disp('+');
  poslin : neural/Transfer Functions/poslin
      settings: SourceProductName=Deep Learning Toolbox  SourceProductBaseCode=NN
  "a{1}" : Outport  InitialOutput=[0;0;0;0;0;0;0;0;0;0;0;0;0;0;0;0;0;0;0;0;0;0;0;0;0;0;0;0;0;0;0;0;0;0;0;0;0;0;0;0;0;0;0;0;0;0;0;0;0;0]
      mask Display: e = 0.06;c1 = [120 230 180]/255;c2 = ([120 230 180]+0)/(256+128);c3 = ([120 230 180]+128)/(256+128);patch([0 1 1 0],[0 0 1 1],c1);patch([0 1 1 1-e 1-e 0+e],[0 0 1 1-e 0+e 0+e],c2);patch([0 0 1 1-e 0+e 0+e],[0 1 1 1-e 1-e 0+e],c3);plot([0 1 1 0 0],[0 0 1 1 0]);
  wires:
  poslin/1 -> "a{1}"/1
  netsum/1 -> poslin/1
  "b{1}"/1 -> netsum/2
  "IW{1,1}"/1("iz{1,1}") -> netsum/1
  Delays 1/1("pd{1,1}") -> "IW{1,1}"/1("pd{1,1}")
  "p{1}"/1 -> Delays 1/1("p{1}")

== system AcasXuClosedLoop_all_comp2017b/networks/Networks_m_9/AcasXu_5_9/Layer 2 ==
  "a{1} " : Inport  PortDimensions=50
      mask Display: e = 0.06;c1 = [120 230 180]/255;c2 = ([120 230 180]+0)/(256+128);c3 = ([120 230 180]+128)/(256+128);patch([0 1 1 0],[0 0 1 1],c1);patch([0 1 1 1-e 1-e 0+e],[0 0 1 1-e 0+e 0+e],c2);patch([0 0 1 1-e 0+e 0+e],[0 1 1 1-e 1-e 0+e],c3);plot([0 1 1 0 0],[0 0 1 1 0]);
  Delays 1 : Subsystem
      mask Display: e = 0.06;c1 = [120 150 230]/255;c2 = ([120 150 230]+0)/383;c3 = ([120 150 230]+128)/383;patch([0 1 1 0],[0 0 1 1],c1);patch([0 1 1 1-e 1-e 0+e],[0 0 1 1-e 0+e 0+e],c2);patch([0 0 1 1-e 0+e 0+e],[0 1 1 1-e 1-e 0+e],c3);plot([0 1 1 0 0],[0 0 1 1 0]);disp('0');
  "LW{2,1}" : Subsystem
      mask Display: e = 0.06;c1 = [120 150 230]/255;c2 = ([120 150 230]+0)/383;c3 = ([120 150 230]+128)/383;patch([0 1 1 0],[0 0 1 1],c1);patch([0 1 1 1-e 1-e 0+e],[0 0 1 1-e 0+e 0+e],c2);patch([0 0 1 1-e 0+e 0+e],[0 1 1 1-e 1-e 0+e],c3);plot([0 1 1 0 0],[0 0 1 1 0]);disp('W');
  "b{2}" : Constant  Value=[-0.373616000000000003655742375485715456306934356689453125;1.4941199999999998926369926266488619148731231689453125;-0.252122000000000012764900247930199839174747467041015625;0.09621200000000000585043125056472490541636943817138671875;-0.9879130000000000411120026910793967545032501220703125;0.017995799999999999296829145123410853557288646697998046875;-0.01507840000000000048319126477736062952317297458648681640625;-0.55142100000000005000089231543825007975101470947265625;-0.1571099999999999996536104163169511593878269195556640625;-0.014368999999999999828137475788025767542421817779541015625;-0.498937000000000019372947690499131567776203155517578125;0.06318769999999999942286166287885862402617931365966796875;0.1557800000000000018030021919912542216479778289794921875;0.485763000000000000344613226843648590147495269775390625;-0.0270981999999999993711252699313263292424380779266357421875;-1.235700000000000020605739337042905390262603759765625;0.52903500000000003300471007605665363371372222900390625;-0.0219647000000000001962430218327426700852811336517333984375;-0.37110500000000001818989403545856475830078125;0.78872299999999995190336221639881841838359832763671875;0.054000399999999997013855335126208956353366374969482421875;-0.0143502999999999998614885754477654700167477130889892578125;-1.6434800000000000519406739840633235871791839599609375;-0.0438411999999999968391506399711943231523036956787109375;1.2733200000000000073896444519050419330596923828125;-0.1544770000000000031992186677598510868847370147705078125;0.1943040000000000044888537331644329242408275604248046875;-0.0147620000000000008155698338896399945951998233795166015625;0.89114400000000004720135393654345534741878509521484375;0.012966800000000000547828449271037243306636810302734375;-0.312985000000000013198331316743860952556133270263671875;0.0311622000000000010100364988829824142158031463623046875;0.43824099999999999166533370953402481973171234130859375;0.70247000000000003883116050928947515785694122314453125;-0.0160894000000000002292832590455873287282884120941162109375;-0.3509320000000000216999751501134596765041351318359375;-0.61837200000000003274891469118301756680011749267578125;0.05627189999999999969748643025013734586536884307861328125;0.66202199999999999935340611045830883085727691650390625;-0.006837010000000000135844668847084903973154723644256591796875;-0.10331200000000000105426778418404865078628063201904296875;1.0944100000000001049471620717667974531650543212890625;0.03349950000000000149835699403411126695573329925537109375;-0.282476000000000004863665026277885772287845611572265625;-1.7712499999999999911182158029987476766109466552734375;-0.059963700000000001721911502272632787935435771942138671875;0.323512999999999995015542708642897196114063262939453125;0.520769000000000037431391319842077791690826416015625;0.1701129999999999864446209585366887040436267852783203125;-0.01518949999999999987687626656907013966701924800872802734375]
      mask Display: e = 0.06;c1 = [120 150 230]/255;c2 = ([120 150 230]+0)/383;c3 = ([120 150 230]+128)/383;patch([0 1 1 0],[0 0 1 1],c1);patch([0 1 1 1-e 1-e 0+e],[0 0 1 1-e 0+e 0+e],c2);patch([0 0 1 1-e 0+e 0+e],[0 1 1 1-e 1-e 0+e],c3);plot([0 1 1 0 0],[0 0 1 1 0]);disp('b');
  netsum : Sum  Inputs=2  SaturateOnIntegerOverflow=on  InputSameDT=on  OutDataTypeStr=Inherit: Same as first input
      mask Display: e = 0.06;c1 = [120 150 230]/255;c2 = ([120 150 230]+0)/383;c3 = ([120 150 230]+128)/383;patch([0 1 1 0],[0 0 1 1],c1);patch([0 1 1 1-e 1-e 0+e],[0 0 1 1-e 0+e 0+e],c2);patch([0 0 1 1-e 0+e 0+e],[0 1 1 1-e 1-e 0+e],c3);plot([0 1 1 0 0],[0 0 1 1 0]);disp('+');
  poslin : neural/Transfer Functions/poslin
      settings: SourceProductName=Deep Learning Toolbox  SourceProductBaseCode=NN
  "a{2}" : Outport  InitialOutput=[0;0;0;0;0;0;0;0;0;0;0;0;0;0;0;0;0;0;0;0;0;0;0;0;0;0;0;0;0;0;0;0;0;0;0;0;0;0;0;0;0;0;0;0;0;0;0;0;0;0]
      mask Display: e = 0.06;c1 = [120 230 180]/255;c2 = ([120 230 180]+0)/(256+128);c3 = ([120 230 180]+128)/(256+128);patch([0 1 1 0],[0 0 1 1],c1);patch([0 1 1 1-e 1-e 0+e],[0 0 1 1-e 0+e 0+e],c2);patch([0 0 1 1-e 0+e 0+e],[0 1 1 1-e 1-e 0+e],c3);plot([0 1 1 0 0],[0 0 1 1 0]);
  wires:
  poslin/1 -> "a{2}"/1
  netsum/1 -> poslin/1
  "b{2}"/1 -> netsum/2
  "LW{2,1}"/1("lz{2,1}") -> netsum/1
  Delays 1/1("pd{2,1}") -> "LW{2,1}"/1("ad{2,1}")
  "a{1} "/1 -> Delays 1/1("p{2}")
